simulink model "Demo_TrafficFlow_cs"  (Simulink 7.6)
settings: Solver=ode5  SolverMode=SingleTasking  StartTime=0.0  StopTime=60  FixedStep=1/20  RelTol=1e-3
PreLoadFcn:
  | check_NUTS_defined
  | try
  |     evalin('base', 'NUTS_defined;');
  | catch
  |     warningMsg = sprintf('PreScan Warning: \nUsing this model requires that MATLAB is configured for PreScan. The model may not display correctly.\n\n ==> Please open MATLAB with the dedicated buttons provided with PreScan');
  |     disp(warningMsg)
  |     warndlg(warningMsg,'WindowStyle','Modal');
  | end
  | 
  | model_closed = 0;
  | assignin('base',['model_closed_' bdroot],false);
InitFcn:
  | PreScanExperimentInitFcn(bdroot)
StopFcn:
  | PreScanExperimentStopFcn(bdroot)
undefined symbols (not defined in the file or in its folder): Advised_Speed_L1, Advised_Speed_L2, Advised_Speed_L3, DiscIntNeverNeededParam, spare_10, spare_5, spare_6, spare_7, spare_8, spare_9, State_L2, Throughput_L1, Throughput_L2, Throughput_L3, Velocity_L1, Velocity_L2, Velocity_L3
libraries: simulink x58, PreScanSimulinkLibrary x40, simulink_extras x32, PreScanUsefulBlocks x22, simulink_need_slupdate x3
(part 11 of 20)

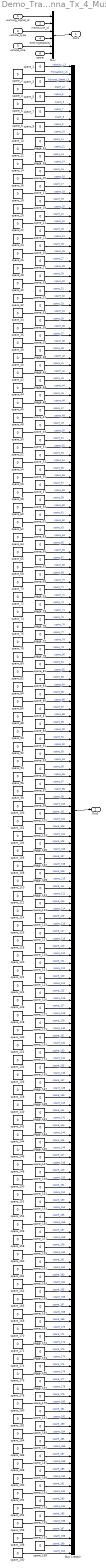
== system Demo_TrafficFlow_cs/HighwayOverhang_3/Antenna_Tx_4_Mux ==
  warning_signal_id : Inport  SampleTime=TimeStep
  maneuvre_id : Inport  Port=2  SampleTime=TimeStep
  vehicle_type : Inport  Port=3  SampleTime=TimeStep
  brakingcapacity : Inport  Port=4  SampleTime=TimeStep
  vehicle_size : Inport  Port=5  SampleTime=TimeStep
  spare : Inport  Port=6  SampleTime=TimeStep
  Bus Creator : BusCreator  Inputs=200
  Mux : Mux  Inputs=6
  spare_{1..200} (200 alike) : Constant  Value=0  SampleTime=TimeStep
  Out1 : Outport
  Out2 : Outport  Port=2  SampleTime=TimeStep
  wires:
  spare_200/1 -> Bus Creator/200  "spare_200"
  spare_199/1 -> Bus Creator/199  "spare_199"
  spare_198/1 -> Bus Creator/198  "spare_198"
  spare_197/1 -> Bus Creator/197  "spare_197"
  spare_196/1 -> Bus Creator/196  "spare_196"
  spare_195/1 -> Bus Creator/195  "spare_195"
  spare_194/1 -> Bus Creator/194  "spare_194"
  spare_193/1 -> Bus Creator/193  "spare_193"
  spare_192/1 -> Bus Creator/192  "spare_192"
  spare_191/1 -> Bus Creator/191  "spare_191"
  spare_190/1 -> Bus Creator/190  "spare_190"
  spare_189/1 -> Bus Creator/189  "spare_189"
  spare_188/1 -> Bus Creator/188  "spare_188"
  spare_187/1 -> Bus Creator/187  "spare_187"
  spare_186/1 -> Bus Creator/186  "spare_186"
  spare_185/1 -> Bus Creator/185  "spare_185"
  spare_184/1 -> Bus Creator/184  "spare_184"
  spare_183/1 -> Bus Creator/183  "spare_183"
  spare_182/1 -> Bus Creator/182  "spare_182"
  spare_181/1 -> Bus Creator/181  "spare_181"
  spare_180/1 -> Bus Creator/180  "spare_180"
  spare_179/1 -> Bus Creator/179  "spare_179"
  spare_178/1 -> Bus Creator/178  "spare_178"
  spare_177/1 -> Bus Creator/177  "spare_177"
  spare_176/1 -> Bus Creator/176  "spare_176"
  spare_175/1 -> Bus Creator/175  "spare_175"
  spare_174/1 -> Bus Creator/174  "spare_174"
  spare_173/1 -> Bus Creator/173  "spare_173"
  spare_172/1 -> Bus Creator/172  "spare_172"
  spare_171/1 -> Bus Creator/171  "spare_171"
  spare_170/1 -> Bus Creator/170  "spare_170"
  spare_169/1 -> Bus Creator/169  "spare_169"
  spare_168/1 -> Bus Creator/168  "spare_168"
  spare_167/1 -> Bus Creator/167  "spare_167"
  spare_166/1 -> Bus Creator/166  "spare_166"
  spare_165/1 -> Bus Creator/165  "spare_165"
  spare_164/1 -> Bus Creator/164  "spare_164"
  spare_163/1 -> Bus Creator/163  "spare_163"
  spare_162/1 -> Bus Creator/162  "spare_162"
  spare_161/1 -> Bus Creator/161  "spare_161"
  spare_160/1 -> Bus Creator/160  "spare_160"
  spare_159/1 -> Bus Creator/159  "spare_159"
  spare_158/1 -> Bus Creator/158  "spare_158"
  spare_157/1 -> Bus Creator/157  "spare_157"
  spare_156/1 -> Bus Creator/156  "spare_156"
  spare_155/1 -> Bus Creator/155  "spare_155"
  spare_154/1 -> Bus Creator/154  "spare_154"
  spare_153/1 -> Bus Creator/153  "spare_153"
  spare_152/1 -> Bus Creator/152  "spare_152"
  spare_151/1 -> Bus Creator/151  "spare_151"
  spare_150/1 -> Bus Creator/150  "spare_150"
  spare_149/1 -> Bus Creator/149  "spare_149"
  spare_148/1 -> Bus Creator/148  "spare_148"
  spare_147/1 -> Bus Creator/147  "spare_147"
  spare_146/1 -> Bus Creator/146  "spare_146"
  spare_145/1 -> Bus Creator/145  "spare_145"
  spare_144/1 -> Bus Creator/144  "spare_144"
  spare_143/1 -> Bus Creator/143  "spare_143"
  spare_142/1 -> Bus Creator/142  "spare_142"
  spare_141/1 -> Bus Creator/141  "spare_141"
  spare_140/1 -> Bus Creator/140  "spare_140"
  spare_139/1 -> Bus Creator/139  "spare_139"
  spare_138/1 -> Bus Creator/138  "spare_138"
  spare_137/1 -> Bus Creator/137  "spare_137"
  spare_136/1 -> Bus Creator/136  "spare_136"
  spare_135/1 -> Bus Creator/135  "spare_135"
  spare_134/1 -> Bus Creator/134  "spare_134"
  spare_133/1 -> Bus Creator/133  "spare_133"
  spare_132/1 -> Bus Creator/132  "spare_132"
  spare_131/1 -> Bus Creator/131  "spare_131"
  spare_130/1 -> Bus Creator/130  "spare_130"
  spare_129/1 -> Bus Creator/129  "spare_129"
  spare_128/1 -> Bus Creator/128  "spare_128"
  spare_127/1 -> Bus Creator/127  "spare_127"
  spare_126/1 -> Bus Creator/126  "spare_126"
  spare_125/1 -> Bus Creator/125  "spare_125"
  spare_124/1 -> Bus Creator/124  "spare_124"
  spare_123/1 -> Bus Creator/123  "spare_123"
  spare_122/1 -> Bus Creator/122  "spare_122"
  spare_121/1 -> Bus Creator/121  "spare_121"
  spare_120/1 -> Bus Creator/120  "spare_120"
  spare_119/1 -> Bus Creator/119  "spare_119"
  spare_118/1 -> Bus Creator/118  "spare_118"
  spare_117/1 -> Bus Creator/117  "spare_117"
  spare_116/1 -> Bus Creator/116  "spare_116"
  spare_115/1 -> Bus Creator/115  "spare_115"
  spare_114/1 -> Bus Creator/114  "spare_114"
  spare_113/1 -> Bus Creator/113  "spare_113"
  spare_112/1 -> Bus Creator/112  "spare_112"
  spare_111/1 -> Bus Creator/111  "spare_111"
  spare_110/1 -> Bus Creator/110  "spare_110"
  spare_109/1 -> Bus Creator/109  "spare_109"
  spare_108/1 -> Bus Creator/108  "spare_108"
  spare_107/1 -> Bus Creator/107  "spare_107"
  spare_106/1 -> Bus Creator/106  "spare_106"
  spare_105/1 -> Bus Creator/105  "spare_105"
  spare_104/1 -> Bus Creator/104  "spare_104"
  spare_103/1 -> Bus Creator/103  "spare_103"
  spare_102/1 -> Bus Creator/102  "spare_102"
  spare_101/1 -> Bus Creator/101  "spare_101"
  spare_100/1 -> Bus Creator/100  "spare_100"
  spare_99/1 -> Bus Creator/99  "spare_99"
  spare_98/1 -> Bus Creator/98  "spare_98"
  spare_97/1 -> Bus Creator/97  "spare_97"
  spare_96/1 -> Bus Creator/96  "spare_96"
  spare_95/1 -> Bus Creator/95  "spare_95"
  spare_94/1 -> Bus Creator/94  "spare_94"
  spare_93/1 -> Bus Creator/93  "spare_93"
  spare_92/1 -> Bus Creator/92  "spare_92"
  spare_91/1 -> Bus Creator/91  "spare_91"
  spare_90/1 -> Bus Creator/90  "spare_90"
  spare_89/1 -> Bus Creator/89  "spare_89"
  spare_88/1 -> Bus Creator/88  "spare_88"
  spare_87/1 -> Bus Creator/87  "spare_87"
  spare_86/1 -> Bus Creator/86  "spare_86"
  spare_85/1 -> Bus Creator/85  "spare_85"
  spare_84/1 -> Bus Creator/84  "spare_84"
  spare_83/1 -> Bus Creator/83  "spare_83"
  spare_82/1 -> Bus Creator/82  "spare_82"
  spare_81/1 -> Bus Creator/81  "spare_81"
  spare_80/1 -> Bus Creator/80  "spare_80"
  spare_79/1 -> Bus Creator/79  "spare_79"
  spare_78/1 -> Bus Creator/78  "spare_78"
  spare_77/1 -> Bus Creator/77  "spare_77"
  spare_76/1 -> Bus Creator/76  "spare_76"
  spare_75/1 -> Bus Creator/75  "spare_75"
  spare_74/1 -> Bus Creator/74  "spare_74"
  spare_73/1 -> Bus Creator/73  "spare_73"
  spare_72/1 -> Bus Creator/72  "spare_72"
  spare_71/1 -> Bus Creator/71  "spare_71"
  spare_70/1 -> Bus Creator/70  "spare_70"
  spare_69/1 -> Bus Creator/69  "spare_69"
  spare_68/1 -> Bus Creator/68  "spare_68"
  spare_67/1 -> Bus Creator/67  "spare_67"
  spare_66/1 -> Bus Creator/66  "spare_66"
  spare_65/1 -> Bus Creator/65  "spare_65"
  spare_64/1 -> Bus Creator/64  "spare_64"
  spare_63/1 -> Bus Creator/63  "spare_63"
  spare_62/1 -> Bus Creator/62  "spare_62"
  spare_61/1 -> Bus Creator/61  "spare_61"
  spare_60/1 -> Bus Creator/60  "spare_60"
  spare_59/1 -> Bus Creator/59  "spare_59"
  spare_58/1 -> Bus Creator/58  "spare_58"
  spare_57/1 -> Bus Creator/57  "spare_57"
  spare_56/1 -> Bus Creator/56  "spare_56"
  spare_55/1 -> Bus Creator/55  "spare_55"
  spare_54/1 -> Bus Creator/54  "spare_54"
  spare_53/1 -> Bus Creator/53  "spare_53"
  spare_52/1 -> Bus Creator/52  "spare_52"
  spare_51/1 -> Bus Creator/51  "spare_51"
  spare_50/1 -> Bus Creator/50  "spare_50"
  spare_49/1 -> Bus Creator/49  "spare_49"
  spare_48/1 -> Bus Creator/48  "spare_48"
  spare_47/1 -> Bus Creator/47  "spare_47"
  spare_46/1 -> Bus Creator/46  "spare_46"
  spare_45/1 -> Bus Creator/45  "spare_45"
  spare_44/1 -> Bus Creator/44  "spare_44"
  spare_43/1 -> Bus Creator/43  "spare_43"
  spare_42/1 -> Bus Creator/42  "spare_42"
  spare_41/1 -> Bus Creator/41  "spare_41"
  spare_40/1 -> Bus Creator/40  "spare_40"
  spare_39/1 -> Bus Creator/39  "spare_39"
  spare_38/1 -> Bus Creator/38  "spare_38"
  spare_37/1 -> Bus Creator/37  "spare_37"
  spare_36/1 -> Bus Creator/36  "spare_36"
  spare_35/1 -> Bus Creator/35  "spare_35"
  spare_34/1 -> Bus Creator/34  "spare_34"
  spare_33/1 -> Bus Creator/33  "spare_33"
  spare_32/1 -> Bus Creator/32  "spare_32"
  spare_31/1 -> Bus Creator/31  "spare_31"
  spare_30/1 -> Bus Creator/30  "spare_30"
  spare_29/1 -> Bus Creator/29  "spare_29"
  spare_28/1 -> Bus Creator/28  "spare_28"
  spare_27/1 -> Bus Creator/27  "spare_27"
  spare_26/1 -> Bus Creator/26  "spare_26"
  spare_25/1 -> Bus Creator/25  "spare_25"
  spare_24/1 -> Bus Creator/24  "spare_24"
  spare_23/1 -> Bus Creator/23  "spare_23"
  spare_22/1 -> Bus Creator/22  "spare_22"
  spare_21/1 -> Bus Creator/21  "spare_21"
  spare_19/1 -> Bus Creator/19  "spare_19"
  spare_18/1 -> Bus Creator/18  "spare_18"
  spare_17/1 -> Bus Creator/17  "spare_17"
  spare_16/1 -> Bus Creator/16  "spare_16"
  spare_15/1 -> Bus Creator/15  "spare_15"
  spare_14/1 -> Bus Creator/14  "spare_14"
  spare_13/1 -> Bus Creator/13  "spare_13"
  spare_12/1 -> Bus Creator/12  "spare_12"
  spare_11/1 -> Bus Creator/11  "spare_11"
  spare_10/1 -> Bus Creator/10  "spare_10"
  spare_9/1 -> Bus Creator/9  "spare_9"
  spare_8/1 -> Bus Creator/8  "spare_8"
  spare_7/1 -> Bus Creator/7  "spare_7"
  spare_6/1 -> Bus Creator/6  "spare_6"
  spare_5/1 -> Bus Creator/5  "spare_5"
  warning_signal_id/1 -> Mux/1
  maneuvre_id/1 -> Mux/2
  vehicle_type/1 -> Mux/3
  brakingcapacity/1 -> Mux/4
  vehicle_size/1 -> Mux/5
  Mux/1 -> Out1/1
  Bus Creator/1 -> Out2/1
  spare/1 -> Mux/6
  spare_1/1 -> Bus Creator/1  "Velocity_L3"
  spare_2/1 -> Bus Creator/2  "Throughput_L3"
  spare_3/1 -> Bus Creator/3  "Advised_Speed_L3"
  spare_4/1 -> Bus Creator/4  "State_L2"
  spare_20/1 -> Bus Creator/20  "spare_20"

== system Demo_TrafficFlow_cs/HighwayOverhang_3/RFBeacon_3_Rx_Demux ==
  Beacon_Rx : Inport
  Demux : Demux  Outputs=19
  Reorder : Subsystem  mask(Output reorder block){NrOfOutputs=19, NrOfObjects=5}
      mask prompts: NrOfOutputs "Number Of Outputs [-]"; NrOfObjects "Number Of Objects [-]"
      mask help: Change output order from object based to output type based
      mask init: same as Demo_TrafficFlow_cs/Audi_A8_1/RFOBU_1_Rx_Demux/Reorder
  compas converter : Subsystem
  OBU_ID : Outport
  Velocity_[m/s] : Outport  Port=2
  Heading [deg] : Outport  Port=3
  vehicle_type : Outport  Port=4
  spare_1 : Outport  Port=5
  spare_2 : Outport  Port=6
  spare_3 : Outport  Port=7
  spare_4 : Outport  Port=8
  spare_5 : Outport  Port=9
  spare_6 : Outport  Port=10
  spare_7 : Outport  Port=11
  spare_8 : Outport  Port=12
  spare_9 : Outport  Port=13
  spare_10 : Outport  Port=14
  spare_11 : Outport  Port=15
  spare_12 : Outport  Port=16
  spare_13 : Outport  Port=17
  spare_14 : Outport  Port=18
  spare_15 : Outport  Port=19
  wires:
  compas converter/1(heading [deg]) -> Heading [deg]/1
  Demux/1 -> OBU_ID/1
  Demux/3 -> compas converter/1(rotz [rad])  "pos_y"
  Demux/2 -> Velocity_[m/s]/1  "pos_x"
  Reorder/1(Out) -> Demux/1
  Demux/5 -> spare_1/1  "pos_z"
  Demux/6 -> spare_2/1  "pos_x"
  Demux/7 -> spare_3/1  "pos_y"
  Demux/8 -> spare_4/1  "pos_z"
  Demux/9 -> spare_5/1
  Demux/10 -> spare_6/1
  Demux/11 -> spare_7/1
  Demux/12 -> spare_8/1
  Demux/13 -> spare_9/1
  Demux/14 -> spare_10/1
  Beacon_Rx/1 -> Reorder/1(In)
  Demux/4 -> vehicle_type/1  "pos_z"
  Demux/15 -> spare_11/1
  Demux/16 -> spare_12/1
  Demux/17 -> spare_13/1
  Demux/18 -> spare_14/1
  Demux/19 -> spare_15/1

== system Demo_TrafficFlow_cs/HighwayOverhang_3/RFBeacon_3_Tx_Mux ==
  BeaconType : Inport
  spare_1 : Inport  Port=2
  spare_2 : Inport  Port=3
  spare_3 : Inport  Port=4
  spare_4 : Inport  Port=5
  spare_5 : Inport  Port=6
  spare_6 : Inport  Port=7
  spare_7 : Inport  Port=8
  spare_8 : Inport  Port=9
  spare_9 : Inport  Port=10
  spare_10 : Inport  Port=11
  spare_11 : Inport  Port=12
  spare_12 : Inport  Port=13
  spare_13 : Inport  Port=14
  spare_14 : Inport  Port=15
  spare_15 : Inport  Port=16
  Mux1 : Mux  Inputs=16
  BeaconTx : Outport
  wires:
  BeaconType/1 -> Mux1/1
  spare_11/1 -> Mux1/12
  spare_12/1 -> Mux1/13
  spare_13/1 -> Mux1/14
  spare_14/1 -> Mux1/15
  spare_15/1 -> Mux1/16
  spare_1/1 -> Mux1/2
  spare_2/1 -> Mux1/3
  spare_3/1 -> Mux1/4
  spare_4/1 -> Mux1/5
  spare_5/1 -> Mux1/6
  spare_6/1 -> Mux1/7
  spare_7/1 -> Mux1/8
  spare_8/1 -> Mux1/9
  spare_9/1 -> Mux1/10
  spare_10/1 -> Mux1/11
  Mux1/1 -> BeaconTx/1

== system Demo_TrafficFlow_cs/HighwayOverhang_3/Throughput detection  Sensor ==
  OBU ID [-] : Inport
  OBU Velocity [m/s] : Inport  Port=2
  Terminator{,1..3} (4 alike) : Terminator
  TrafficDensityCounter [cars/hour] : Subsystem  mask{MT=5}
      mask prompts: MT "Monitor Time"
      mask Display: color('red');text(5,10,['MT=',num2str(MT)])
  MeanVelocity [km/h] : Outport
  Throughput [#/min] : Outport  Port=2
  wires:
  OBU ID [-]/1 -> TrafficDensityCounter [cars/hour]/1(Detection)
  TrafficDensityCounter [cars/hour]/1(Out_[1/MT]_unweighted) -> Terminator/1  "TP [1/MT unweighted]"
  TrafficDensityCounter [cars/hour]/2(Out_[1/MT]) -> Terminator1/1  "TP [1/MT]"
  TrafficDensityCounter [cars/hour]/3(Out_[1/s]) -> Terminator2/1  "TP [1/s]"
  TrafficDensityCounter [cars/hour]/4(Out_[1/min]) -> Throughput [#/min]/1  "TP [1/min]"
  TrafficDensityCounter [cars/hour]/5(Out_[1/hour]) -> Terminator3/1  "TP [1/hour]"
  TrafficDensityCounter [cars/hour]/6(Velocity [km/h]) -> MeanVelocity [km/h]/1  "velocity [km/h]"
  OBU Velocity [m/s]/1 -> TrafficDensityCounter [cars/hour]/2(Velocity [m/s])

== system Demo_TrafficFlow_cs/Mazda_RX8_1/AIR_4_Demux ==
  AIR : Inport
  Activate_Switch : Subsystem
      mask Display: text(5,10,'')
  Bus Selector : BusSelector  OutputSignals=DATA.Range,DATA.Azimuth,DATA.Elevation,DATA.ID,DATA.Velocity,DATA.Heading
  Data Type Conversion : DataTypeConversion  OutDataTypeStr=double
  For Iterator : ForIterator  ResetStates=reset  IterationLimit=detectableObjects
  Selector : Selector  InputPortWidth=detectableObjects  IndexOptions=Index vector (port)  Indices=[1 3]  OutputSizes=1
  Signal2ArrayOfSignals1 : Subsystem  mask{VECTORLENGTH=detectableObjects}
      mask prompts: VECTORLENGTH "Length of vector"
  Signal2ArrayOfSignals2 : Subsystem  mask{VECTORLENGTH=detectableObjects}
      mask prompts: VECTORLENGTH "Length of vector"
  Signal2ArrayOfSignals3 : Subsystem  mask{VECTORLENGTH=detectableObjects}
      mask prompts: VECTORLENGTH "Length of vector"
  Signal2ArrayOfSignals4 : Subsystem  mask{VECTORLENGTH=detectableObjects}
      mask prompts: VECTORLENGTH "Length of vector"
  Signal2ArrayOfSignals5 : Subsystem  mask{VECTORLENGTH=detectableObjects}
      mask prompts: VECTORLENGTH "Length of vector"
  Signal2ArrayOfSignals6 : Subsystem  mask{VECTORLENGTH=detectableObjects}
      mask prompts: VECTORLENGTH "Length of vector"
  Range [m] : Outport
  Azimuth [deg] : Outport  Port=2
  Elevation [deg] : Outport  Port=3
  ID [-] : Outport  Port=4
  Velocity [m/s] : Outport  Port=5
  Heading [deg] : Outport  Port=6
  wires:
  Data Type Conversion/1 -> ID [-]/1
  Bus Selector/6 -> Signal2ArrayOfSignals6/2(Element)  "<Heading>"
  Signal2ArrayOfSignals6/1(ArrayofElements) -> Activate_Switch/2(Heading)
  Bus Selector/5 -> Signal2ArrayOfSignals5/2(Element)  "<Velocity>"
  Signal2ArrayOfSignals5/1(ArrayofElements) -> Velocity [m/s]/1
  Bus Selector/4 -> Signal2ArrayOfSignals4/2(Element)  "<ID>"
  Signal2ArrayOfSignals4/1(ArrayofElements) -> Data Type Conversion/1, Activate_Switch/1(BeamID)
  Bus Selector/3 -> Signal2ArrayOfSignals3/2(Element)  "<Elevation>"
  Signal2ArrayOfSignals3/1(ArrayofElements) -> Elevation [deg]/1
  Bus Selector/2 -> Signal2ArrayOfSignals1/2(Element)  "<Azimuth>"
  Signal2ArrayOfSignals1/1(ArrayofElements) -> Azimuth [deg]/1
  Signal2ArrayOfSignals2/1(ArrayofElements) -> Range [m]/1
  Bus Selector/1 -> Signal2ArrayOfSignals2/2(Element)  "<Range>"
  Selector/1 -> Bus Selector/1
  For Iterator/1 -> Signal2ArrayOfSignals6/1(LoopIndex), Signal2ArrayOfSignals5/1(LoopIndex), Signal2ArrayOfSignals4/1(LoopIndex), Signal2ArrayOfSignals3/1(LoopIndex), Signal2ArrayOfSignals1/1(LoopIndex), Signal2ArrayOfSignals2/1(LoopIndex), Selector/2
  AIR/1 -> Selector/1
  Activate_Switch/1(On/Off) -> Heading [deg]/1

== system Demo_TrafficFlow_cs/Mazda_RX8_1/RFOBU_4_Rx_Demux ==
  Beacon_Rx : Inport
  Demux1 : Demux  Outputs=3
  Demux2 : Demux  Outputs=20
  Mux : Mux  Inputs=3
  Reorder : Subsystem  mask(Output reorder block){NrOfOutputs=20, NrOfObjects=2}
      mask prompts: NrOfOutputs "Number Of Outputs [-]"; NrOfObjects "Number Of Objects [-]"
      mask help: Change output order from object based to output type based
      mask init: same as Demo_TrafficFlow_cs/Audi_A8_1/RFOBU_1_Rx_Demux/Reorder
  xyz2GPS : PreScanUsefulBlocks/xyz2GPS  CoordinateCount=2  GPS_base=[0.000000 0.000000 0.000]
  Beacon_ID : Outport
  GPS_pos_lat_[deg] : Outport  Port=2
  GPS_pos_long_[deg] : Outport  Port=3
  GPS_pos_alt_[m] : Outport  Port=4
  BeaconType [-] : Outport  Port=5
  spare_1 : Outport  Port=6
  spare_2 : Outport  Port=7
  spare_3 : Outport  Port=8
  spare_4 : Outport  Port=9
  spare_5 : Outport  Port=10
  spare_6 : Outport  Port=11
  spare_7 : Outport  Port=12
  spare_8 : Outport  Port=13
  spare_9 : Outport  Port=14
  spare_10 : Outport  Port=15
  spare_11 : Outport  Port=16
  spare_12 : Outport  Port=17
  spare_13 : Outport  Port=18
  spare_14 : Outport  Port=19
  spare_15 : Outport  Port=20
  wires:
  Demux2/5 -> BeaconType [-]/1  "pos_z"
  Mux/1 -> xyz2GPS/1
  Demux2/20 -> spare_15/1
  Demux2/19 -> spare_14/1
  Demux2/18 -> spare_13/1
  Demux2/17 -> spare_12/1
  Demux2/16 -> spare_11/1
  Beacon_Rx/1 -> Reorder/1(In)
  Demux2/15 -> spare_10/1
  Demux2/14 -> spare_9/1
  Demux2/13 -> spare_8/1
  Demux2/12 -> spare_7/1
  Demux2/11 -> spare_6/1
  Demux2/10 -> spare_5/1
  Demux2/9 -> spare_4/1
  Demux2/8 -> spare_3/1
  Demux2/7 -> spare_2/1
  Demux2/6 -> spare_1/1  "pos_z"
  Reorder/1(Out) -> Demux2/1
  Demux1/3 -> GPS_pos_alt_[m]/1
  Demux1/2 -> GPS_pos_long_[deg]/1
  Demux1/1 -> GPS_pos_lat_[deg]/1
  xyz2GPS/1 -> Demux1/1
  Demux2/4 -> Mux/3  "pos_z"
  Demux2/3 -> Mux/2  "pos_y"
  Demux2/2 -> Mux/1  "pos_x"
  Demux2/1 -> Beacon_ID/1

== system Demo_TrafficFlow_cs/Mazda_RX8_1/RFOBU_4_Tx_Mux ==
  Vehicle_Type : Inport
  spare_1 : Inport  Port=2
  spare_2 : Inport  Port=3
  spare_3 : Inport  Port=4
  spare_4 : Inport  Port=5
  spare_5 : Inport  Port=6
  spare_6 : Inport  Port=7
  spare_7 : Inport  Port=8
  spare_8 : Inport  Port=9
  spare_9 : Inport  Port=10
  spare_10 : Inport  Port=11
  spare_11 : Inport  Port=12
  spare_12 : Inport  Port=13
  spare_13 : Inport  Port=14
  spare_14 : Inport  Port=15
  spare_15 : Inport  Port=16
  Mux1 : Mux  Inputs=16
  BeaconTx : Outport
  wires:
  Vehicle_Type/1 -> Mux1/1
  Mux1/1 -> BeaconTx/1
  spare_10/1 -> Mux1/11
  spare_9/1 -> Mux1/10
  spare_8/1 -> Mux1/9
  spare_7/1 -> Mux1/8
  spare_6/1 -> Mux1/7
  spare_5/1 -> Mux1/6
  spare_4/1 -> Mux1/5
  spare_3/1 -> Mux1/4
  spare_2/1 -> Mux1/3
  spare_1/1 -> Mux1/2
  spare_15/1 -> Mux1/16
  spare_14/1 -> Mux1/15
  spare_13/1 -> Mux1/14
  spare_12/1 -> Mux1/13
  spare_11/1 -> Mux1/12

== system Demo_TrafficFlow_cs/Mazda_RX8_1/SELF_Demux ==
  SELF : Inport
  Demux1 : Demux  Outputs=3
  Demux2 : Demux  Outputs=3
  Demux3 : Demux  Outputs=[3 3 1 1]
  Demux4 : Demux  Outputs=3
  Gain : Gain  Gain=180/pi
  Gain1 : Gain  Gain=180/pi
  Selector : Selector  InputPortWidth=3  IndexOptions=Index vector (dialog)  Indices=[3]  OutputSizes=1
  compas converter : Subsystem
  xyz2GPS : PreScanUsefulBlocks/xyz2GPS  CoordinateCount=1  GPS_base=[0.000000 0.000000 0.000]
  x [m] : Outport
  y [m] : Outport  Port=2
  z [m] : Outport  Port=3
  rotx [deg] : Outport  Port=4
  roty [deg] : Outport  Port=5
  rotz [deg] : Outport  Port=6
  GPS lat [deg] : Outport  Port=7
  GPS long [deg] : Outport  Port=8
  GPS alt [m] : Outport  Port=9
  velocity [m/s] : Outport  Port=10
  heading [deg] : Outport  Port=11
  yaw rate [deg/s] : Outport  Port=12
  wires:
  Demux3/4 -> Gain1/1
  Gain1/1 -> yaw rate [deg/s]/1
  Demux3/3 -> velocity [m/s]/1
  compas converter/1(heading [deg]) -> heading [deg]/1
  xyz2GPS/1 -> Demux4/1
  Demux4/3 -> GPS alt [m]/1
  Demux4/2 -> GPS long [deg]/1
  Demux4/1 -> GPS lat [deg]/1
  Demux3/2 -> Gain/1, Selector/1
  Demux3/1 -> xyz2GPS/1, Demux1/1
  SELF/1 -> Demux3/1
  Demux2/3 -> rotz [deg]/1
  Demux2/2 -> roty [deg]/1
  Demux2/1 -> rotx [deg]/1
  Demux1/3 -> z [m]/1
  Demux1/2 -> y [m]/1
  Demux1/1 -> x [m]/1
  Gain/1 -> Demux2/1
  Selector/1 -> compas converter/1(rotz [rad])

== system Demo_TrafficFlow_cs/Mazda_RX8_1/Simple_Longitudinal_ACC ==
  note: v_ini=50 km/h
  Advised Velocity [km/h] : Inport
  Range [m] : Inport  Port=2
  Velocity [m/s] : Inport  Port=3
  HOLD Advised Speed : Subsystem
  HardCoded Safety Distance [m] : Constant  Value=15
  LONGITUDINAL ACC & Dynamics : Subsystem
  Manual Switch : simulink/Signal Routing/Manual Switch  SystemSampleTime=-1  sw=1  action=0  varsize=off
  Manual Switch1 : simulink/Signal Routing/Manual Switch  SystemSampleTime=-1  sw=1  action=0  varsize=off
  Memory2 : Memory
  Own Speed Intention [km/h] : Constant  Value=60
  Product1 : Product  RndMeth=Zero
  TimeToCollision [s] Velocity Dependent Safety Distance : Constant
  ACC_Velocity [m/s] : Outport
  wires:
  Range [m]/1 -> LONGITUDINAL ACC & Dynamics/1(Detected Range [m])
  TimeToCollision [s] Velocity Dependent Safety Distance/1 -> Product1/1
  HardCoded Safety Distance [m]/1 -> Manual Switch/1
  Product1/1 -> Manual Switch/2
  Manual Switch/1 -> LONGITUDINAL ACC & Dynamics/4(Safety Distance [m])
  Advised Velocity [km/h]/1 -> HOLD Advised Speed/1(v)
  Own Speed Intention [km/h]/1 -> Manual Switch1/2
  HOLD Advised Speed/1(v_hold) -> Manual Switch1/1
  Manual Switch1/1 -> LONGITUDINAL ACC & Dynamics/3(Own Velocity Intention [km/h])
  LONGITUDINAL ACC & Dynamics/1(Velocity [m/s]) -> ACC_Velocity [m/s]/1, Memory2/1
  Velocity [m/s]/1 -> LONGITUDINAL ACC & Dynamics/2(Detected Velocity Vehicle [m/s])
  Memory2/1 -> Product1/2

== system Demo_TrafficFlow_cs/Mazda_RX8_1/Trajectories ==
  Velocity : Inport
  OBUID_Reinsert [-] : Inport  Port=2
  StateLANE2 [-] : Inport  Port=3
  END OF TRACK REACHED & GO TO BUFFER : Subsystem
  ElapsedTrack1 : Subsystem  Tag=ElapsedTrack  mask(PreScan Demux Block:){Filename='PathFollowerSva_4_Path.mat'}
      mask prompts: Filename "Name path-file"
      mask init:
      | route=load(['trajectories/',Filename]);
      | 
      | s=route.path(1,:);
      | x=route.path(2,:);
      | y=route.path(3,:);
      | z=route.path(4,:);
      | roll=route.path(5,:);
      | pitch=route.path(6,:);
      | yaw=route.path(7,:);
      settings: DeleteFcn=PreScanBlockDeleteFcn;
  Integrator : Integrator  ExternalReset=level  InitialConditionSource=external  LimitOutput=on  UpperSaturationLimit=180  LowerSaturationLimit=-1e-3
  Integrator1 : DiscreteIntegrator  Tag=TrajIntegrator  SampleTime=1/20  ICPrevOutput=DiscIntNeverNeededParam  ICPrevScaledInput=DiscIntNeverNeededParam
  LANE CHANGE LEFT (cummulative y position;  multiple shift to left are possible) : Subsystem  mask{Filename='Path_LC_Left.mat'}
      mask prompts: Filename "Name path-file"
      mask init:
      | %route=load(['Trajectories/',Filename]);
      | route=load(Filename);
      | s=route.path(1,:);
      | x=route.path(2,:);
      | y=route.path(3,:);
      | yaw=route.path(4,:);
  Lane 1 : Constant
  Length_of_Track [m] : Constant  Value=180
  OBU_ID : Constant  Value=25
  PathFollowerSva_4 : Subsystem  Tag=Trajectory  mask(PreScan Source Block: Trajectory){FileName='PathFollowerSva_4.mat', TimeStep=1/20}
      mask prompts: TimeStep "Time Step [s]"
      mask help:
      | The Trajectory block contains the trajectories defined in the PreScan Experiment Editor. The output of the trajectory block is the (vehicle) State.
      |
      | For more information, please click Help.
      mask init: same as Demo_TrafficFlow_cs/Audi_A8_1/Trajectories/PathFollowerSva_2
      settings: DeleteFcn=PreScanBlockDeleteFcn;
      mask Help: eval('openHelp(312)')
      mask Display: fprintf(FileName)
  PathFollowerSva_4_Offset : Constant  Tag=TrajectoryOffset  Value="[0  0  0  0  0  0]"
  Product : Product  RndMeth=Zero
  Product1 : Product  RndMeth=Zero
  RESTART TRAJECT : Subsystem
  Random  LC generator : Subsystem
  Relational Operator2 : RelationalOperator  Operator===  OutDataTypeStr=boolean
  Selector1 : Constant  Tag=TrajSubSelector  Value=2
  Step : Step  Time=0.01  Before=1  After=0  SampleTime=0
  Sum : Sum  Inputs=|+-
  Sum2 : Sum  Inputs=|++
  Terminator_1 : Terminator
  Traj2State1 : Subsystem  Tag=Traj2State
      settings: DeleteFcn=PreScanBlockDeleteFcn;
      mask Type: PreScan Demux Block:
  TrajSwitch1 : MultiPortSwitch  Tag=TrajSubSwitch  Inputs=2  zeroidx=off
  Velocity1 : Constant  Tag=TrajVelocity  Value=15
  x0 : Constant  Value=100
  State : Outport  Tag=TrajOutport
  wires:
  END OF TRACK REACHED & GO TO BUFFER/1(Buffer_offset [m]) -> Sum/2
  LANE CHANGE LEFT (cummulative y position;  multiple shift to left are possible)/1(cum LC) -> Sum2/2  "Add Lane Change"
  Sum/1 -> Sum2/1
  RESTART TRAJECT/1(reset [-]) -> [reset]
  END OF TRACK REACHED & GO TO BUFFER/2(End_of_Track_Reached [-]) -> RESTART TRAJECT/1(End_of_Track_Reached [-])
  Step/1 -> Product/1
  x0/1 -> Product/2
  OBUID_Reinsert [-]/1 -> RESTART TRAJECT/2(ID_start_again [-])
  OBU_ID/1 -> RESTART TRAJECT/3(objectID [-])
  Lane 1/1 -> Relational Operator2/2
  [reset] -> LANE CHANGE LEFT (cummulative y position;  multiple shift to left are possible)/1(reset cum value)
  Relational Operator2/1 -> LANE CHANGE LEFT (cummulative y position;  multiple shift to left are possible)/3(on/off [-])
  Length_of_Track [m]/1 -> END OF TRACK REACHED & GO TO BUFFER/2(Length_of_Track [m])
  Random  LC generator/1(0/1) -> Product1/2
  Product1/1 -> Relational Operator2/1
  StateLANE2 [-]/1 -> Product1/1
  RESTART TRAJECT/2(Start_Traject [-]) -> Integrator/2
  Product/1 -> Integrator/3
  Velocity/1 -> Integrator/1, LANE CHANGE LEFT (cummulative y position;  multiple shift to left are possible)/2(velocity [m/s])
  Integrator/1 -> END OF TRACK REACHED & GO TO BUFFER/1(Elapsed_Track [m]), ElapsedTrack1/2(s)
  PathFollowerSva_4/1(trajectory) -> Traj2State1/1(Traj Org)
  PathFollowerSva_4_Offset/1 -> Traj2State1/2(Offset), ElapsedTrack1/1(Offset)
  Velocity1/1 -> Integrator1/1
  Selector1/1 -> TrajSwitch1/1
  Traj2State1/1(state) -> TrajSwitch1/2
  ElapsedTrack1/1(state) -> Sum/1
  TrajSwitch1/1 -> State/1
  Sum2/1 -> TrajSwitch1/3
  Integrator1/1 -> Terminator_1/1

== system Demo_TrafficFlow_cs/Motorcyclist_1/AIR_5_Demux ==
  AIR : Inport
  Activate_Switch : Subsystem
      mask Display: text(5,10,'')
  Bus Selector : BusSelector  OutputSignals=DATA.Range,DATA.Azimuth,DATA.Elevation,DATA.ID,DATA.Velocity,DATA.Heading
  Data Type Conversion : DataTypeConversion  OutDataTypeStr=double
  For Iterator : ForIterator  ResetStates=reset  IterationLimit=detectableObjects
  Selector : Selector  InputPortWidth=detectableObjects  IndexOptions=Index vector (port)  Indices=[1 3]  OutputSizes=1
  Signal2ArrayOfSignals1 : Subsystem  mask{VECTORLENGTH=detectableObjects}
      mask prompts: VECTORLENGTH "Length of vector"
  Signal2ArrayOfSignals2 : Subsystem  mask{VECTORLENGTH=detectableObjects}
      mask prompts: VECTORLENGTH "Length of vector"
  Signal2ArrayOfSignals3 : Subsystem  mask{VECTORLENGTH=detectableObjects}
      mask prompts: VECTORLENGTH "Length of vector"
  Signal2ArrayOfSignals4 : Subsystem  mask{VECTORLENGTH=detectableObjects}
      mask prompts: VECTORLENGTH "Length of vector"
  Signal2ArrayOfSignals5 : Subsystem  mask{VECTORLENGTH=detectableObjects}
      mask prompts: VECTORLENGTH "Length of vector"
  Signal2ArrayOfSignals6 : Subsystem  mask{VECTORLENGTH=detectableObjects}
      mask prompts: VECTORLENGTH "Length of vector"
  Range [m] : Outport
  Azimuth [deg] : Outport  Port=2
  Elevation [deg] : Outport  Port=3
  ID [-] : Outport  Port=4
  Velocity [m/s] : Outport  Port=5
  Heading [deg] : Outport  Port=6
  wires:
  Data Type Conversion/1 -> ID [-]/1
  Bus Selector/6 -> Signal2ArrayOfSignals6/2(Element)  "<Heading>"
  Signal2ArrayOfSignals6/1(ArrayofElements) -> Activate_Switch/2(Heading)
  Bus Selector/5 -> Signal2ArrayOfSignals5/2(Element)  "<Velocity>"
  Signal2ArrayOfSignals5/1(ArrayofElements) -> Velocity [m/s]/1
  Bus Selector/4 -> Signal2ArrayOfSignals4/2(Element)  "<ID>"
  Signal2ArrayOfSignals4/1(ArrayofElements) -> Data Type Conversion/1, Activate_Switch/1(BeamID)
  Bus Selector/3 -> Signal2ArrayOfSignals3/2(Element)  "<Elevation>"
  Signal2ArrayOfSignals3/1(ArrayofElements) -> Elevation [deg]/1
  Bus Selector/2 -> Signal2ArrayOfSignals1/2(Element)  "<Azimuth>"
  Signal2ArrayOfSignals1/1(ArrayofElements) -> Azimuth [deg]/1
  Signal2ArrayOfSignals2/1(ArrayofElements) -> Range [m]/1
  Bus Selector/1 -> Signal2ArrayOfSignals2/2(Element)  "<Range>"
  Selector/1 -> Bus Selector/1
  For Iterator/1 -> Signal2ArrayOfSignals6/1(LoopIndex), Signal2ArrayOfSignals5/1(LoopIndex), Signal2ArrayOfSignals4/1(LoopIndex), Signal2ArrayOfSignals3/1(LoopIndex), Signal2ArrayOfSignals1/1(LoopIndex), Signal2ArrayOfSignals2/1(LoopIndex), Selector/2
  AIR/1 -> Selector/1
  Activate_Switch/1(On/Off) -> Heading [deg]/1

== system Demo_TrafficFlow_cs/Motorcyclist_1/RFOBU_5_Rx_Demux ==
  Beacon_Rx : Inport
  Demux1 : Demux  Outputs=3
  Demux2 : Demux  Outputs=20
  Mux : Mux  Inputs=3
  Reorder : Subsystem  mask(Output reorder block){NrOfOutputs=20, NrOfObjects=2}
      mask prompts: NrOfOutputs "Number Of Outputs [-]"; NrOfObjects "Number Of Objects [-]"
      mask help: Change output order from object based to output type based
      mask init: same as Demo_TrafficFlow_cs/Audi_A8_1/RFOBU_1_Rx_Demux/Reorder
  xyz2GPS : PreScanUsefulBlocks/xyz2GPS  CoordinateCount=2  GPS_base=[0.000000 0.000000 0.000]
  Beacon_ID : Outport
  GPS_pos_lat_[deg] : Outport  Port=2
  GPS_pos_long_[deg] : Outport  Port=3
  GPS_pos_alt_[m] : Outport  Port=4
  BeaconType [-] : Outport  Port=5
  spare_1 : Outport  Port=6
  spare_2 : Outport  Port=7
  spare_3 : Outport  Port=8
  spare_4 : Outport  Port=9
  spare_5 : Outport  Port=10
  spare_6 : Outport  Port=11
  spare_7 : Outport  Port=12
  spare_8 : Outport  Port=13
  spare_9 : Outport  Port=14
  spare_10 : Outport  Port=15
  spare_11 : Outport  Port=16
  spare_12 : Outport  Port=17
  spare_13 : Outport  Port=18
  spare_14 : Outport  Port=19
  spare_15 : Outport  Port=20
  wires:
  Demux2/5 -> BeaconType [-]/1  "pos_z"
  Mux/1 -> xyz2GPS/1
  Demux2/20 -> spare_15/1
  Demux2/19 -> spare_14/1
  Demux2/18 -> spare_13/1
  Demux2/17 -> spare_12/1
  Demux2/16 -> spare_11/1
  Beacon_Rx/1 -> Reorder/1(In)
  Demux2/15 -> spare_10/1
  Demux2/14 -> spare_9/1
  Demux2/13 -> spare_8/1
  Demux2/12 -> spare_7/1
  Demux2/11 -> spare_6/1
  Demux2/10 -> spare_5/1
  Demux2/9 -> spare_4/1
  Demux2/8 -> spare_3/1
  Demux2/7 -> spare_2/1
  Demux2/6 -> spare_1/1  "pos_z"
  Reorder/1(Out) -> Demux2/1
  Demux1/3 -> GPS_pos_alt_[m]/1
  Demux1/2 -> GPS_pos_long_[deg]/1
  Demux1/1 -> GPS_pos_lat_[deg]/1
  xyz2GPS/1 -> Demux1/1
  Demux2/4 -> Mux/3  "pos_z"
  Demux2/3 -> Mux/2  "pos_y"
  Demux2/2 -> Mux/1  "pos_x"
  Demux2/1 -> Beacon_ID/1

== system Demo_TrafficFlow_cs/Motorcyclist_1/RFOBU_5_Tx_Mux ==
  Vehicle_Type : Inport
  spare_1 : Inport  Port=2
  spare_2 : Inport  Port=3
  spare_3 : Inport  Port=4
  spare_4 : Inport  Port=5
  spare_5 : Inport  Port=6
  spare_6 : Inport  Port=7
  spare_7 : Inport  Port=8
  spare_8 : Inport  Port=9
  spare_9 : Inport  Port=10
  spare_10 : Inport  Port=11
  spare_11 : Inport  Port=12
  spare_12 : Inport  Port=13
  spare_13 : Inport  Port=14
  spare_14 : Inport  Port=15
  spare_15 : Inport  Port=16
  Mux1 : Mux  Inputs=16
  BeaconTx : Outport
  wires:
  Vehicle_Type/1 -> Mux1/1
  Mux1/1 -> BeaconTx/1
  spare_10/1 -> Mux1/11
  spare_9/1 -> Mux1/10
  spare_8/1 -> Mux1/9
  spare_7/1 -> Mux1/8
  spare_6/1 -> Mux1/7
  spare_5/1 -> Mux1/6
  spare_4/1 -> Mux1/5
  spare_3/1 -> Mux1/4
  spare_2/1 -> Mux1/3
  spare_1/1 -> Mux1/2
  spare_15/1 -> Mux1/16
  spare_14/1 -> Mux1/15
  spare_13/1 -> Mux1/14
  spare_12/1 -> Mux1/13
  spare_11/1 -> Mux1/12

== system Demo_TrafficFlow_cs/Motorcyclist_1/SELF_Demux ==
  SELF : Inport
  Demux1 : Demux  Outputs=3
  Demux2 : Demux  Outputs=3
  Demux3 : Demux  Outputs=[3 3 1 1]
  Demux4 : Demux  Outputs=3
  Gain : Gain  Gain=180/pi
  Gain1 : Gain  Gain=180/pi
  Selector : Selector  InputPortWidth=3  IndexOptions=Index vector (dialog)  Indices=[3]  OutputSizes=1
  compas converter : Subsystem
  xyz2GPS : PreScanUsefulBlocks/xyz2GPS  CoordinateCount=1  GPS_base=[0.000000 0.000000 0.000]
  x [m] : Outport
  y [m] : Outport  Port=2
  z [m] : Outport  Port=3
  rotx [deg] : Outport  Port=4
  roty [deg] : Outport  Port=5
  rotz [deg] : Outport  Port=6
  GPS lat [deg] : Outport  Port=7
  GPS long [deg] : Outport  Port=8
  GPS alt [m] : Outport  Port=9
  velocity [m/s] : Outport  Port=10
  heading [deg] : Outport  Port=11
  yaw rate [deg/s] : Outport  Port=12
  wires:
  Demux3/4 -> Gain1/1
  Gain1/1 -> yaw rate [deg/s]/1
  Demux3/3 -> velocity [m/s]/1
  compas converter/1(heading [deg]) -> heading [deg]/1
  xyz2GPS/1 -> Demux4/1
  Demux4/3 -> GPS alt [m]/1
  Demux4/2 -> GPS long [deg]/1
  Demux4/1 -> GPS lat [deg]/1
  Demux3/2 -> Gain/1, Selector/1
  Demux3/1 -> xyz2GPS/1, Demux1/1
  SELF/1 -> Demux3/1
  Demux2/3 -> rotz [deg]/1
  Demux2/2 -> roty [deg]/1
  Demux2/1 -> rotx [deg]/1
  Demux1/3 -> z [m]/1
  Demux1/2 -> y [m]/1
  Demux1/1 -> x [m]/1
  Gain/1 -> Demux2/1
  Selector/1 -> compas converter/1(rotz [rad])

== system Demo_TrafficFlow_cs/Motorcyclist_1/Simple_Longitudinal_ACC ==
  note: v_ini=50 km/h
  Advised Velocity [km/h] : Inport
  Range [m] : Inport  Port=2
  Velocity [m/s] : Inport  Port=3
  HOLD Advised Speed : Subsystem
  HardCoded Safety Distance [m] : Constant  Value=15
  LONGITUDINAL ACC & Dynamics : Subsystem
  Manual Switch : simulink/Signal Routing/Manual Switch  SystemSampleTime=-1  sw=1  action=0  varsize=off
  Manual Switch1 : simulink/Signal Routing/Manual Switch  SystemSampleTime=-1  sw=0  action=0  varsize=off
  Memory2 : Memory
  Own Speed Intention [km/h] : Constant  Value=80
  Product1 : Product  RndMeth=Zero
  TimeToCollision [s] Velocity Dependent Safety Distance : Constant
  ACC_Velocity [m/s] : Outport
  wires:
  Range [m]/1 -> LONGITUDINAL ACC & Dynamics/1(Detected Range [m])
  TimeToCollision [s] Velocity Dependent Safety Distance/1 -> Product1/1
  HardCoded Safety Distance [m]/1 -> Manual Switch/1
  Product1/1 -> Manual Switch/2
  Manual Switch/1 -> LONGITUDINAL ACC & Dynamics/4(Safety Distance [m])
  Advised Velocity [km/h]/1 -> HOLD Advised Speed/1(v)
  Own Speed Intention [km/h]/1 -> Manual Switch1/2
  HOLD Advised Speed/1(v_hold) -> Manual Switch1/1
  Manual Switch1/1 -> LONGITUDINAL ACC & Dynamics/3(Own Velocity Intention [km/h])
  LONGITUDINAL ACC & Dynamics/1(Velocity [m/s]) -> ACC_Velocity [m/s]/1, Memory2/1
  Velocity [m/s]/1 -> LONGITUDINAL ACC & Dynamics/2(Detected Velocity Vehicle [m/s])
  Memory2/1 -> Product1/2

== system Demo_TrafficFlow_cs/Motorcyclist_1/Trajectories ==
  Velocity : Inport
  OBUID_Reinsert [-] : Inport  Port=2
  StateLANE2 [-] : Inport  Port=3
  END OF TRACK REACHED & GO TO BUFFER : Subsystem
  ElapsedTrack1 : Subsystem  Tag=ElapsedTrack  mask(PreScan Demux Block:){Filename='PathFollowerSva_5_Path.mat'}
      mask prompts: Filename "Name path-file"
      mask init:
      | route=load(['trajectories/',Filename]);
      | 
      | s=route.path(1,:);
      | x=route.path(2,:);
      | y=route.path(3,:);
      | z=route.path(4,:);
      | roll=route.path(5,:);
      | pitch=route.path(6,:);
      | yaw=route.path(7,:);
      settings: DeleteFcn=PreScanBlockDeleteFcn;
  Integrator : Integrator  ExternalReset=level  InitialConditionSource=external  LimitOutput=on  UpperSaturationLimit=180  LowerSaturationLimit=-1e-3
  Integrator1 : DiscreteIntegrator  Tag=TrajIntegrator  SampleTime=1/20  ICPrevOutput=DiscIntNeverNeededParam  ICPrevScaledInput=DiscIntNeverNeededParam
  LANE CHANGE LEFT (cummulative y position;  multiple shift to left are possible) : Subsystem  mask{Filename='Path_LC_Left.mat'}
      mask prompts: Filename "Name path-file"
      mask init:
      | %route=load(['Trajectories/',Filename]);
      | route=load(Filename);
      | s=route.path(1,:);
      | x=route.path(2,:);
      | y=route.path(3,:);
      | yaw=route.path(4,:);
  Lane 1 : Constant
  Length_of_Track [m] : Constant  Value=180
  OBU_ID : Constant  Value=26
  PathFollowerSva_5 : Subsystem  Tag=Trajectory  mask(PreScan Source Block: Trajectory){FileName='PathFollowerSva_5.mat', TimeStep=1/20}
      mask prompts: TimeStep "Time Step [s]"
      mask help:
      | The Trajectory block contains the trajectories defined in the PreScan Experiment Editor. The output of the trajectory block is the (vehicle) State.
      |
      | For more information, please click Help.
      mask init: same as Demo_TrafficFlow_cs/Audi_A8_1/Trajectories/PathFollowerSva_2
      settings: DeleteFcn=PreScanBlockDeleteFcn;
      mask Help: eval('openHelp(312)')
      mask Display: fprintf(FileName)
  PathFollowerSva_5_Offset : Constant  Tag=TrajectoryOffset  Value="[0  0  0  0  0  0]"
  Product : Product  RndMeth=Zero
  Product1 : Product  RndMeth=Zero
  RESTART TRAJECT : Subsystem
  Random  LC generator : Subsystem
  Relational Operator2 : RelationalOperator  Operator===  OutDataTypeStr=boolean
  Selector1 : Constant  Tag=TrajSubSelector  Value=2
  Step : Step  Time=0.01  Before=1  After=0  SampleTime=0
  Sum : Sum  Inputs=|+-
  Sum2 : Sum  Inputs=|++
  Terminator_1 : Terminator
  Traj2State1 : Subsystem  Tag=Traj2State
      settings: DeleteFcn=PreScanBlockDeleteFcn;
      mask Type: PreScan Demux Block:
  TrajSwitch1 : MultiPortSwitch  Tag=TrajSubSwitch  Inputs=2  zeroidx=off
  Velocity1 : Constant  Tag=TrajVelocity  Value=15
  x0 : Constant  Value=160
  State : Outport  Tag=TrajOutport
  wires:
  END OF TRACK REACHED & GO TO BUFFER/1(Buffer_offset [m]) -> Sum/2
  LANE CHANGE LEFT (cummulative y position;  multiple shift to left are possible)/1(cum LC) -> Sum2/2  "Add Lane Change"
  Sum/1 -> Sum2/1
  RESTART TRAJECT/1(reset [-]) -> [reset]
  END OF TRACK REACHED & GO TO BUFFER/2(End_of_Track_Reached [-]) -> RESTART TRAJECT/1(End_of_Track_Reached [-])
  Step/1 -> Product/1
  x0/1 -> Product/2
  OBUID_Reinsert [-]/1 -> RESTART TRAJECT/2(ID_start_again [-])
  OBU_ID/1 -> RESTART TRAJECT/3(objectID [-])
  Lane 1/1 -> Relational Operator2/2
  [reset] -> LANE CHANGE LEFT (cummulative y position;  multiple shift to left are possible)/1(reset cum value)
  Relational Operator2/1 -> LANE CHANGE LEFT (cummulative y position;  multiple shift to left are possible)/3(on/off [-])
  Length_of_Track [m]/1 -> END OF TRACK REACHED & GO TO BUFFER/2(Length_of_Track [m])
  Random  LC generator/1(0/1) -> Product1/2
  Product1/1 -> Relational Operator2/1
  StateLANE2 [-]/1 -> Product1/1
  RESTART TRAJECT/2(Start_Traject [-]) -> Integrator/2
  Product/1 -> Integrator/3
  Velocity/1 -> Integrator/1, LANE CHANGE LEFT (cummulative y position;  multiple shift to left are possible)/2(velocity [m/s])
  Integrator/1 -> END OF TRACK REACHED & GO TO BUFFER/1(Elapsed_Track [m]), ElapsedTrack1/2(s)
  PathFollowerSva_5/1(trajectory) -> Traj2State1/1(Traj Org)
  PathFollowerSva_5_Offset/1 -> Traj2State1/2(Offset), ElapsedTrack1/1(Offset)
  Velocity1/1 -> Integrator1/1
  Selector1/1 -> TrajSwitch1/1
  Traj2State1/1(state) -> TrajSwitch1/2
  ElapsedTrack1/1(state) -> Sum/1
  TrajSwitch1/1 -> State/1
  Sum2/1 -> TrajSwitch1/3
  Integrator1/1 -> Terminator_1/1

== system Demo_TrafficFlow_cs/Nissan_Cabstar_1/AIR_6_Demux ==
  AIR : Inport
  Activate_Switch : Subsystem
      mask Display: text(5,10,'')
  Bus Selector : BusSelector  OutputSignals=DATA.Range,DATA.Azimuth,DATA.Elevation,DATA.ID,DATA.Velocity,DATA.Heading
  Data Type Conversion : DataTypeConversion  OutDataTypeStr=double
  For Iterator : ForIterator  ResetStates=reset  IterationLimit=detectableObjects
  Selector : Selector  InputPortWidth=detectableObjects  IndexOptions=Index vector (port)  Indices=[1 3]  OutputSizes=1
  Signal2ArrayOfSignals1 : Subsystem  mask{VECTORLENGTH=detectableObjects}
      mask prompts: VECTORLENGTH "Length of vector"
  Signal2ArrayOfSignals2 : Subsystem  mask{VECTORLENGTH=detectableObjects}
      mask prompts: VECTORLENGTH "Length of vector"
  Signal2ArrayOfSignals3 : Subsystem  mask{VECTORLENGTH=detectableObjects}
      mask prompts: VECTORLENGTH "Length of vector"
  Signal2ArrayOfSignals4 : Subsystem  mask{VECTORLENGTH=detectableObjects}
      mask prompts: VECTORLENGTH "Length of vector"
  Signal2ArrayOfSignals5 : Subsystem  mask{VECTORLENGTH=detectableObjects}
      mask prompts: VECTORLENGTH "Length of vector"
  Signal2ArrayOfSignals6 : Subsystem  mask{VECTORLENGTH=detectableObjects}
      mask prompts: VECTORLENGTH "Length of vector"
  Range [m] : Outport
  Azimuth [deg] : Outport  Port=2
  Elevation [deg] : Outport  Port=3
  ID [-] : Outport  Port=4
  Velocity [m/s] : Outport  Port=5
  Heading [deg] : Outport  Port=6
  wires:
  Data Type Conversion/1 -> ID [-]/1
  Bus Selector/6 -> Signal2ArrayOfSignals6/2(Element)  "<Heading>"
  Signal2ArrayOfSignals6/1(ArrayofElements) -> Activate_Switch/2(Heading)
  Bus Selector/5 -> Signal2ArrayOfSignals5/2(Element)  "<Velocity>"
  Signal2ArrayOfSignals5/1(ArrayofElements) -> Velocity [m/s]/1
  Bus Selector/4 -> Signal2ArrayOfSignals4/2(Element)  "<ID>"
  Signal2ArrayOfSignals4/1(ArrayofElements) -> Data Type Conversion/1, Activate_Switch/1(BeamID)
  Bus Selector/3 -> Signal2ArrayOfSignals3/2(Element)  "<Elevation>"
  Signal2ArrayOfSignals3/1(ArrayofElements) -> Elevation [deg]/1
  Bus Selector/2 -> Signal2ArrayOfSignals1/2(Element)  "<Azimuth>"
  Signal2ArrayOfSignals1/1(ArrayofElements) -> Azimuth [deg]/1
  Signal2ArrayOfSignals2/1(ArrayofElements) -> Range [m]/1
  Bus Selector/1 -> Signal2ArrayOfSignals2/2(Element)  "<Range>"
  Selector/1 -> Bus Selector/1
  For Iterator/1 -> Signal2ArrayOfSignals6/1(LoopIndex), Signal2ArrayOfSignals5/1(LoopIndex), Signal2ArrayOfSignals4/1(LoopIndex), Signal2ArrayOfSignals3/1(LoopIndex), Signal2ArrayOfSignals1/1(LoopIndex), Signal2ArrayOfSignals2/1(LoopIndex), Selector/2
  AIR/1 -> Selector/1
  Activate_Switch/1(On/Off) -> Heading [deg]/1

== system Demo_TrafficFlow_cs/Nissan_Cabstar_1/RFOBU_6_Rx_Demux ==
  Beacon_Rx : Inport
  Demux1 : Demux  Outputs=3
  Demux2 : Demux  Outputs=20
  Mux : Mux  Inputs=3
  Reorder : Subsystem  mask(Output reorder block){NrOfOutputs=20, NrOfObjects=2}
      mask prompts: NrOfOutputs "Number Of Outputs [-]"; NrOfObjects "Number Of Objects [-]"
      mask help: Change output order from object based to output type based
      mask init: same as Demo_TrafficFlow_cs/Audi_A8_1/RFOBU_1_Rx_Demux/Reorder
  xyz2GPS : PreScanUsefulBlocks/xyz2GPS  CoordinateCount=2  GPS_base=[0.000000 0.000000 0.000]
  Beacon_ID : Outport
  GPS_pos_lat_[deg] : Outport  Port=2
  GPS_pos_long_[deg] : Outport  Port=3
  GPS_pos_alt_[m] : Outport  Port=4
  BeaconType [-] : Outport  Port=5
  spare_1 : Outport  Port=6
  spare_2 : Outport  Port=7
  spare_3 : Outport  Port=8
  spare_4 : Outport  Port=9
  spare_5 : Outport  Port=10
  spare_6 : Outport  Port=11
  spare_7 : Outport  Port=12
  spare_8 : Outport  Port=13
  spare_9 : Outport  Port=14
  spare_10 : Outport  Port=15
  spare_11 : Outport  Port=16
  spare_12 : Outport  Port=17
  spare_13 : Outport  Port=18
  spare_14 : Outport  Port=19
  spare_15 : Outport  Port=20
  wires:
  Demux2/5 -> BeaconType [-]/1  "pos_z"
  Mux/1 -> xyz2GPS/1
  Demux2/20 -> spare_15/1
  Demux2/19 -> spare_14/1
  Demux2/18 -> spare_13/1
  Demux2/17 -> spare_12/1
  Demux2/16 -> spare_11/1
  Beacon_Rx/1 -> Reorder/1(In)
  Demux2/15 -> spare_10/1
  Demux2/14 -> spare_9/1
  Demux2/13 -> spare_8/1
  Demux2/12 -> spare_7/1
  Demux2/11 -> spare_6/1
  Demux2/10 -> spare_5/1
  Demux2/9 -> spare_4/1
  Demux2/8 -> spare_3/1
  Demux2/7 -> spare_2/1
  Demux2/6 -> spare_1/1  "pos_z"
  Reorder/1(Out) -> Demux2/1
  Demux1/3 -> GPS_pos_alt_[m]/1
  Demux1/2 -> GPS_pos_long_[deg]/1
  Demux1/1 -> GPS_pos_lat_[deg]/1
  xyz2GPS/1 -> Demux1/1
  Demux2/4 -> Mux/3  "pos_z"
  Demux2/3 -> Mux/2  "pos_y"
  Demux2/2 -> Mux/1  "pos_x"
  Demux2/1 -> Beacon_ID/1

== system Demo_TrafficFlow_cs/Nissan_Cabstar_1/RFOBU_6_Tx_Mux ==
  Vehicle_Type : Inport
  spare_1 : Inport  Port=2
  spare_2 : Inport  Port=3
  spare_3 : Inport  Port=4
  spare_4 : Inport  Port=5
  spare_5 : Inport  Port=6
  spare_6 : Inport  Port=7
  spare_7 : Inport  Port=8
  spare_8 : Inport  Port=9
  spare_9 : Inport  Port=10
  spare_10 : Inport  Port=11
  spare_11 : Inport  Port=12
  spare_12 : Inport  Port=13
  spare_13 : Inport  Port=14
  spare_14 : Inport  Port=15
  spare_15 : Inport  Port=16
  Mux1 : Mux  Inputs=16
  BeaconTx : Outport
  wires:
  Vehicle_Type/1 -> Mux1/1
  Mux1/1 -> BeaconTx/1
  spare_10/1 -> Mux1/11
  spare_9/1 -> Mux1/10
  spare_8/1 -> Mux1/9
  spare_7/1 -> Mux1/8
  spare_6/1 -> Mux1/7
  spare_5/1 -> Mux1/6
  spare_4/1 -> Mux1/5
  spare_3/1 -> Mux1/4
  spare_2/1 -> Mux1/3
  spare_1/1 -> Mux1/2
  spare_15/1 -> Mux1/16
  spare_14/1 -> Mux1/15
  spare_13/1 -> Mux1/14
  spare_12/1 -> Mux1/13
  spare_11/1 -> Mux1/12

== system Demo_TrafficFlow_cs/Nissan_Cabstar_1/SELF_Demux ==
  SELF : Inport
  Demux1 : Demux  Outputs=3
  Demux2 : Demux  Outputs=3
  Demux3 : Demux  Outputs=[3 3 1 1]
  Demux4 : Demux  Outputs=3
  Gain : Gain  Gain=180/pi
  Gain1 : Gain  Gain=180/pi
  Selector : Selector  InputPortWidth=3  IndexOptions=Index vector (dialog)  Indices=[3]  OutputSizes=1
  compas converter : Subsystem
  xyz2GPS : PreScanUsefulBlocks/xyz2GPS  CoordinateCount=1  GPS_base=[0.000000 0.000000 0.000]
  x [m] : Outport
  y [m] : Outport  Port=2
  z [m] : Outport  Port=3
  rotx [deg] : Outport  Port=4
  roty [deg] : Outport  Port=5
  rotz [deg] : Outport  Port=6
  GPS lat [deg] : Outport  Port=7
  GPS long [deg] : Outport  Port=8
  GPS alt [m] : Outport  Port=9
  velocity [m/s] : Outport  Port=10
  heading [deg] : Outport  Port=11
  yaw rate [deg/s] : Outport  Port=12
  wires:
  Demux3/4 -> Gain1/1
  Gain1/1 -> yaw rate [deg/s]/1
  Demux3/3 -> velocity [m/s]/1
  compas converter/1(heading [deg]) -> heading [deg]/1
  xyz2GPS/1 -> Demux4/1
  Demux4/3 -> GPS alt [m]/1
  Demux4/2 -> GPS long [deg]/1
  Demux4/1 -> GPS lat [deg]/1
  Demux3/2 -> Gain/1, Selector/1
  Demux3/1 -> xyz2GPS/1, Demux1/1
  SELF/1 -> Demux3/1
  Demux2/3 -> rotz [deg]/1
  Demux2/2 -> roty [deg]/1
  Demux2/1 -> rotx [deg]/1
  Demux1/3 -> z [m]/1
  Demux1/2 -> y [m]/1
  Demux1/1 -> x [m]/1
  Gain/1 -> Demux2/1
  Selector/1 -> compas converter/1(rotz [rad])
